simulink model "cddDcmApp"  (R2022b)
settings: Solver=FixedStepDiscrete  StartTime=0.0  StopTime=10.0  FixedStep=0.02  RelTol=1e-3
undefined symbols (not defined in the file or in its folder): CCPFaultType, DcChlType, HFAChlType, IcuChannelType, MtrRoutStaEnum, P_SignalPartType, P_Std_ReturnType, PowerChlType, SrsAdChlType, VGAChlType
(part 3 of 13)

== system cddDcmApp/DataServices_DID_D962_DID_D962_2_No_Of_Movements ==
  DataServices_DID_D962_DID_D962_2_No_Of_Movements_ReadData_sys : Subsystem
  DataServices_DID_D962_DID_D962_2_No_Of_Movements_WriteData_sys : Subsystem

== system cddDcmApp/DataServices_DID_D963_ComplexStructure_2 ==
  DataServices_DID_D963_ComplexStructure_2_ReadData_sys : Subsystem

== system cddDcmApp/DataServices_DID_D963_DID_D963_1_Requested_Position ==
  DataServices_DID_D963_DID_D963_1_Requested_Position_ReadData_sys : Subsystem

== system cddDcmApp/DataServices_DID_D963_DID_D963_2_Actual_Position ==
  DataServices_DID_D963_DID_D963_2_Actual_Position_ReadData_sys : Subsystem

== system cddDcmApp/DataServices_DID_D964_DID_D964_1_step_Range ==
  DataServices_DID_D964_DID_D964_1_step_Range_ReadData_sys : Subsystem
  DataServices_DID_D964_DID_D964_1_step_Range_WriteData_sys : Subsystem

== system cddDcmApp/DataServices_DID_D964_DID_D964_2_No_Of_Movements ==
  DataServices_DID_D964_DID_D964_2_No_Of_Movements_ReadData_sys : Subsystem
  DataServices_DID_D964_DID_D964_2_No_Of_Movements_WriteData_sys : Subsystem

== system cddDcmApp/DataServices_DID_D965_ComplexStructure_2 ==
  DataServices_DID_D965_ComplexStructure_2_ReadData_sys : Subsystem

== system cddDcmApp/DataServices_DID_D965_DID_D965_1_Requested_Position ==
  DataServices_DID_D965_DID_D965_1_Requested_Position_ReadData_sys : Subsystem

== system cddDcmApp/DataServices_DID_D965_DID_D965_2_Actual_Position ==
  DataServices_DID_D965_DID_D965_2_Actual_Position_ReadData_sys : Subsystem

== system cddDcmApp/DataServices_DID_D966_DID_D966_1_step_Range ==
  DataServices_DID_D966_DID_D966_1_step_Range_ReadData_sys : Subsystem
  DataServices_DID_D966_DID_D966_1_step_Range_WriteData_sys : Subsystem

== system cddDcmApp/DataServices_DID_D966_DID_D966_2_No_Of_Movements ==
  DataServices_DID_D966_DID_D966_2_No_Of_Movements_ReadData_sys : Subsystem
  DataServices_DID_D966_DID_D966_2_No_Of_Movements_WriteData_sys : Subsystem

== system cddDcmApp/DataServices_DID_D967_ComplexStructure_2 ==
  DataServices_DID_D967_ComplexStructure_2_ReadData_sys : Subsystem

== system cddDcmApp/DataServices_DID_D967_DID_D967_1_Requested_Position ==
  DataServices_DID_D967_DID_D967_1_Requested_Position_ReadData_sys : Subsystem

== system cddDcmApp/DataServices_DID_D967_DID_D967_2_Actual_Position ==
  DataServices_DID_D967_DID_D967_2_Actual_Position_ReadData_sys : Subsystem

== system cddDcmApp/DataServices_DID_D968_DID_D968_1_step_Range ==
  DataServices_DID_D968_DID_D968_1_step_Range_ReadData_sys : Subsystem
  DataServices_DID_D968_DID_D968_1_step_Range_WriteData_sys : Subsystem

== system cddDcmApp/DataServices_DID_D968_DID_D968_2_No_Of_Movements ==
  DataServices_DID_D968_DID_D968_2_No_Of_Movements_ReadData_sys : Subsystem
  DataServices_DID_D968_DID_D968_2_No_Of_Movements_WriteData_sys : Subsystem

== system cddDcmApp/DataServices_DID_D969_ComplexStructure_2 ==
  DataServices_DID_D969_ComplexStructure_2_ReadData_sys : Subsystem

== system cddDcmApp/DataServices_DID_D969_DID_D969_1_Requested_Position ==
  DataServices_DID_D969_DID_D969_1_Requested_Position_ReadData_sys : Subsystem

== system cddDcmApp/DataServices_DID_D969_DID_D969_2_Actual_Position ==
  DataServices_DID_D969_DID_D969_2_Actual_Position_ReadData_sys : Subsystem

== system cddDcmApp/DataServices_DID_D970_Stepper_Motor_12_Mode_Panel_First_Left_Flap_DID_D970_1_step_Range ==
  DataServices_DID_D970_Stepper_Motor_12_Mode_Panel_First_Left_Flap_DID_D970_1_step_Range_ConditionCheckRead_sys : Subsystem
  DataServices_DID_D970_Stepper_Motor_12_Mode_Panel_First_Left_Flap_DID_D970_1_step_Range_ReadData_sys : Subsystem
  DataServices_DID_D970_Stepper_Motor_12_Mode_Panel_First_Left_Flap_DID_D970_1_step_Range_WriteData_sys : Subsystem

== system cddDcmApp/DataServices_DID_D970_Stepper_Motor_12_Mode_Panel_First_Left_Flap_DID_D970_2_No_Of_Movements ==
  DataServices_DID_D970_Stepper_Motor_12_Mode_Panel_First_Left_Flap_DID_D970_2_No_Of_Movements_ConditionCheckRead_sys : Subsystem
  DataServices_DID_D970_Stepper_Motor_12_Mode_Panel_First_Left_Flap_DID_D970_2_No_Of_Movements_ReadData_sys : Subsystem
  DataServices_DID_D970_Stepper_Motor_12_Mode_Panel_First_Left_Flap_DID_D970_2_No_Of_Movements_WriteData_sys : Subsystem

== system cddDcmApp/DataServices_DID_D971_Stepper_Motor_12_Mode_Panel_First_Left_Flap_position_ComplexStructure_2 ==
  DataServices_DID_D971_Stepper_Motor_12_Mode_Panel_First_Left_Flap_position_ComplexStructure_2_ConditionCheckRead_sys : Subsystem
  DataServices_DID_D971_Stepper_Motor_12_Mode_Panel_First_Left_Flap_position_ComplexStructure_2_ReadData_sys : Subsystem

== system cddDcmApp/DataServices_DID_D971_Stepper_Motor_12_Mode_Panel_First_Left_Flap_position_DID_D971_1_Requested_Position ==
  DataServices_DID_D971_Stepper_Motor_12_Mode_Panel_First_Left_Flap_position_DID_D971_1_Requested_Position_ConditionCheckRead_sys : Subsystem
  DataServices_DID_D971_Stepper_Motor_12_Mode_Panel_First_Left_Flap_position_DID_D971_1_Requested_Position_ReadData_sys : Subsystem

== system cddDcmApp/DataServices_DID_D971_Stepper_Motor_12_Mode_Panel_First_Left_Flap_position_DID_D971_2_Actual_Position ==
  DataServices_DID_D971_Stepper_Motor_12_Mode_Panel_First_Left_Flap_position_DID_D971_2_Actual_Position_ConditionCheckRead_sys : Subsystem
  DataServices_DID_D971_Stepper_Motor_12_Mode_Panel_First_Left_Flap_position_DID_D971_2_Actual_Position_ReadData_sys : Subsystem

== system cddDcmApp/DataServices_DID_D972_Stepper_Motor_13_Mode_Panel_First_Side_Left_Flap_DID_D972_1_step_Range ==
  DataServices_DID_D972_Stepper_Motor_13_Mode_Panel_First_Side_Left_Flap_DID_D972_1_step_Range_ConditionCheckRead_sys : Subsystem
  DataServices_DID_D972_Stepper_Motor_13_Mode_Panel_First_Side_Left_Flap_DID_D972_1_step_Range_ReadData_sys : Subsystem
  DataServices_DID_D972_Stepper_Motor_13_Mode_Panel_First_Side_Left_Flap_DID_D972_1_step_Range_WriteData_sys : Subsystem

== system cddDcmApp/DataServices_DID_D972_Stepper_Motor_13_Mode_Panel_First_Side_Left_Flap_DID_D972_2_No_Of_Movements ==
  DataServices_DID_D972_Stepper_Motor_13_Mode_Panel_First_Side_Left_Flap_DID_D972_2_No_Of_Movements_ConditionCheckRead_sys : Subsystem
  DataServices_DID_D972_Stepper_Motor_13_Mode_Panel_First_Side_Left_Flap_DID_D972_2_No_Of_Movements_ReadData_sys : Subsystem
  DataServices_DID_D972_Stepper_Motor_13_Mode_Panel_First_Side_Left_Flap_DID_D972_2_No_Of_Movements_WriteData_sys : Subsystem

== system cddDcmApp/DataServices_DID_D973_Stepper_Motor_13_Mode_Panel_First_Side_Left_Flap_position_ComplexStructure_2 ==
  DataServices_DID_D973_Stepper_Motor_13_Mode_Panel_First_Side_Left_Flap_position_ComplexStructure_2_ConditionCheckRead_sys : Subsystem
  DataServices_DID_D973_Stepper_Motor_13_Mode_Panel_First_Side_Left_Flap_position_ComplexStructure_2_ReadData_sys : Subsystem

== system cddDcmApp/DataServices_DID_D973_Stepper_Motor_13_Mode_Panel_First_Side_Left_Flap_position_DID_D973_1_Requested_Position ==
  DataServices_DID_D973_Stepper_Motor_13_Mode_Panel_First_Side_Left_Flap_position_DID_D973_1_Requested_Position_ConditionCheckRead_sys : Subsystem
  DataServices_DID_D973_Stepper_Motor_13_Mode_Panel_First_Side_Left_Flap_position_DID_D973_1_Requested_Position_ReadData_sys : Subsystem

== system cddDcmApp/DataServices_DID_D973_Stepper_Motor_13_Mode_Panel_First_Side_Left_Flap_position_DID_D973_2_Actual_Position ==
  DataServices_DID_D973_Stepper_Motor_13_Mode_Panel_First_Side_Left_Flap_position_DID_D973_2_Actual_Position_ConditionCheckRead_sys : Subsystem
  DataServices_DID_D973_Stepper_Motor_13_Mode_Panel_First_Side_Left_Flap_position_DID_D973_2_Actual_Position_ReadData_sys : Subsystem

== system cddDcmApp/DataServices_DID_D974_Stepper_Motor_14_Mode_Panel_First_Right_Flap_DID_D974_1_step_Range ==
  DataServices_DID_D974_Stepper_Motor_14_Mode_Panel_First_Right_Flap_DID_D974_1_step_Range_ConditionCheckRead_sys : Subsystem
  DataServices_DID_D974_Stepper_Motor_14_Mode_Panel_First_Right_Flap_DID_D974_1_step_Range_ReadData_sys : Subsystem
  DataServices_DID_D974_Stepper_Motor_14_Mode_Panel_First_Right_Flap_DID_D974_1_step_Range_WriteData_sys : Subsystem

== system cddDcmApp/DataServices_DID_D974_Stepper_Motor_14_Mode_Panel_First_Right_Flap_DID_D974_2_No_Of_Movements ==
  DataServices_DID_D974_Stepper_Motor_14_Mode_Panel_First_Right_Flap_DID_D974_2_No_Of_Movements_ConditionCheckRead_sys : Subsystem
  DataServices_DID_D974_Stepper_Motor_14_Mode_Panel_First_Right_Flap_DID_D974_2_No_Of_Movements_ReadData_sys : Subsystem
  DataServices_DID_D974_Stepper_Motor_14_Mode_Panel_First_Right_Flap_DID_D974_2_No_Of_Movements_WriteData_sys : Subsystem

== system cddDcmApp/DataServices_DID_D975_Stepper_Motor_14_Mode_Panel_First_Right_Flap_position_ComplexStructure_2 ==
  DataServices_DID_D975_Stepper_Motor_14_Mode_Panel_First_Right_Flap_position_ComplexStructure_2_ConditionCheckRead_sys : Subsystem
  DataServices_DID_D975_Stepper_Motor_14_Mode_Panel_First_Right_Flap_position_ComplexStructure_2_ReadData_sys : Subsystem

== system cddDcmApp/DataServices_DID_D975_Stepper_Motor_14_Mode_Panel_First_Right_Flap_position_DID_D975_1_Requested_Position ==
  DataServices_DID_D975_Stepper_Motor_14_Mode_Panel_First_Right_Flap_position_DID_D975_1_Requested_Position_ConditionCheckRead_sys : Subsystem
  DataServices_DID_D975_Stepper_Motor_14_Mode_Panel_First_Right_Flap_position_DID_D975_1_Requested_Position_ReadData_sys : Subsystem

== system cddDcmApp/DataServices_DID_D975_Stepper_Motor_14_Mode_Panel_First_Right_Flap_position_DID_D975_2_Actual_Position ==
  DataServices_DID_D975_Stepper_Motor_14_Mode_Panel_First_Right_Flap_position_DID_D975_2_Actual_Position_ConditionCheckRead_sys : Subsystem
  DataServices_DID_D975_Stepper_Motor_14_Mode_Panel_First_Right_Flap_position_DID_D975_2_Actual_Position_ReadData_sys : Subsystem

== system cddDcmApp/DataServices_DID_D976_Stepper_Motor_15_Mode_Panel_First_Side_Right_Flap_DID_D976_1_step_Range ==
  DataServices_DID_D976_Stepper_Motor_15_Mode_Panel_First_Side_Right_Flap_DID_D976_1_step_Range_ConditionCheckRead_sys : Subsystem
  DataServices_DID_D976_Stepper_Motor_15_Mode_Panel_First_Side_Right_Flap_DID_D976_1_step_Range_ReadData_sys : Subsystem
  DataServices_DID_D976_Stepper_Motor_15_Mode_Panel_First_Side_Right_Flap_DID_D976_1_step_Range_WriteData_sys : Subsystem

== system cddDcmApp/DataServices_DID_D976_Stepper_Motor_15_Mode_Panel_First_Side_Right_Flap_DID_D976_2_No_Of_Movements ==
  DataServices_DID_D976_Stepper_Motor_15_Mode_Panel_First_Side_Right_Flap_DID_D976_2_No_Of_Movements_ConditionCheckRead_sys : Subsystem
  DataServices_DID_D976_Stepper_Motor_15_Mode_Panel_First_Side_Right_Flap_DID_D976_2_No_Of_Movements_ReadData_sys : Subsystem
  DataServices_DID_D976_Stepper_Motor_15_Mode_Panel_First_Side_Right_Flap_DID_D976_2_No_Of_Movements_WriteData_sys : Subsystem

== system cddDcmApp/DataServices_DID_D977_Stepper_Motor_15_Mode_Panel_First_Side_Right_Flap_position_ComplexStructure_2 ==
  DataServices_DID_D977_Stepper_Motor_15_Mode_Panel_First_Side_Right_Flap_position_ComplexStructure_2_ConditionCheckRead_sys : Subsystem
  DataServices_DID_D977_Stepper_Motor_15_Mode_Panel_First_Side_Right_Flap_position_ComplexStructure_2_ReadData_sys : Subsystem

== system cddDcmApp/DataServices_DID_D977_Stepper_Motor_15_Mode_Panel_First_Side_Right_Flap_position_DID_D977_1_Requested_Position ==
  DataServices_DID_D977_Stepper_Motor_15_Mode_Panel_First_Side_Right_Flap_position_DID_D977_1_Requested_Position_ReadData_sys : Subsystem

== system cddDcmApp/DataServices_DID_D977_Stepper_Motor_15_Mode_Panel_First_Side_Right_Flap_position_DID_D977_2_Actual_Position ==
  DataServices_DID_D977_Stepper_Motor_15_Mode_Panel_First_Side_Right_Flap_position_DID_D977_2_Actual_Position_ConditionCheckRead_sys : Subsystem
  DataServices_DID_D977_Stepper_Motor_15_Mode_Panel_First_Side_Right_Flap_position_DID_D977_2_Actual_Position_ReadData_sys : Subsystem

== system cddDcmApp/DataServices_DID_D978_Stepper_Motor_16_Mode_Floor_First_Left_Flap_DID_D978_1_step_Range ==
  DataServices_DID_D978_Stepper_Motor_16_Mode_Floor_First_Left_Flap_DID_D978_1_step_Range_ConditionCheckRead_sys : Subsystem
  DataServices_DID_D978_Stepper_Motor_16_Mode_Floor_First_Left_Flap_DID_D978_1_step_Range_ReadData_sys : Subsystem
  DataServices_DID_D978_Stepper_Motor_16_Mode_Floor_First_Left_Flap_DID_D978_1_step_Range_WriteData_sys : Subsystem

== system cddDcmApp/DataServices_DID_D978_Stepper_Motor_16_Mode_Floor_First_Left_Flap_DID_D978_2_No_Of_Movements ==
  DataServices_DID_D978_Stepper_Motor_16_Mode_Floor_First_Left_Flap_DID_D978_2_No_Of_Movements_ConditionCheckRead_sys : Subsystem
  DataServices_DID_D978_Stepper_Motor_16_Mode_Floor_First_Left_Flap_DID_D978_2_No_Of_Movements_ReadData_sys : Subsystem
  DataServices_DID_D978_Stepper_Motor_16_Mode_Floor_First_Left_Flap_DID_D978_2_No_Of_Movements_WriteData_sys : Subsystem

== system cddDcmApp/DataServices_DID_D979_Stepper_Motor_16_Mode_Floor_First_Left_Flap_position_ComplexStructure_2 ==
  DataServices_DID_D979_Stepper_Motor_16_Mode_Floor_First_Left_Flap_position_ComplexStructure_2_ConditionCheckRead_sys : Subsystem
  DataServices_DID_D979_Stepper_Motor_16_Mode_Floor_First_Left_Flap_position_ComplexStructure_2_ReadData_sys : Subsystem

== system cddDcmApp/DataServices_DID_D979_Stepper_Motor_16_Mode_Floor_First_Left_Flap_position_DID_D979_1_Requested_Position ==
  DataServices_DID_D979_Stepper_Motor_16_Mode_Floor_First_Left_Flap_position_DID_D979_1_Requested_Position_ConditionCheckRead_sys : Subsystem
  DataServices_DID_D979_Stepper_Motor_16_Mode_Floor_First_Left_Flap_position_DID_D979_1_Requested_Position_ReadData_sys : Subsystem

== system cddDcmApp/DataServices_DID_D979_Stepper_Motor_16_Mode_Floor_First_Left_Flap_position_DID_D979_2_Actual_Position ==
  DataServices_DID_D979_Stepper_Motor_16_Mode_Floor_First_Left_Flap_position_DID_D979_2_Actual_Position_ConditionCheckRead_sys : Subsystem
  DataServices_DID_D979_Stepper_Motor_16_Mode_Floor_First_Left_Flap_position_DID_D979_2_Actual_Position_ReadData_sys : Subsystem

== system cddDcmApp/DataServices_DID_D97A_Stepper_Motor_17_Mode_Floor_First_Right_Flap_DID_D97A_1_step_Range ==
  DataServices_DID_D97A_Stepper_Motor_17_Mode_Floor_First_Right_Flap_DID_D97A_1_step_Range_ConditionCheckRead_sys : Subsystem
  DataServices_DID_D97A_Stepper_Motor_17_Mode_Floor_First_Right_Flap_DID_D97A_1_step_Range_ReadData_sys : Subsystem
  DataServices_DID_D97A_Stepper_Motor_17_Mode_Floor_First_Right_Flap_DID_D97A_1_step_Range_WriteData_sys : Subsystem

== system cddDcmApp/DataServices_DID_D97A_Stepper_Motor_17_Mode_Floor_First_Right_Flap_DID_D97A_2_No_Of_Movements ==
  DataServices_DID_D97A_Stepper_Motor_17_Mode_Floor_First_Right_Flap_DID_D97A_2_No_Of_Movements_ConditionCheckRead_sys : Subsystem
  DataServices_DID_D97A_Stepper_Motor_17_Mode_Floor_First_Right_Flap_DID_D97A_2_No_Of_Movements_ReadData_sys : Subsystem
  DataServices_DID_D97A_Stepper_Motor_17_Mode_Floor_First_Right_Flap_DID_D97A_2_No_Of_Movements_WriteData_sys : Subsystem

== system cddDcmApp/DataServices_DID_D97B_Stepper_Motor_17_Mode_Floor_First_Right_Flap_position_ComplexStructure_2 ==
  DataServices_DID_D97B_Stepper_Motor_17_Mode_Floor_First_Right_Flap_position_ComplexStructure_2_ConditionCheckRead_sys : Subsystem
  DataServices_DID_D97B_Stepper_Motor_17_Mode_Floor_First_Right_Flap_position_ComplexStructure_2_ReadData_sys : Subsystem

== system cddDcmApp/DataServices_DID_D97B_Stepper_Motor_17_Mode_Floor_First_Right_Flap_position_DID_D97B_1_Requested_Position ==
  DataServices_DID_D97B_Stepper_Motor_17_Mode_Floor_First_Right_Flap_position_DID_D97B_1_Requested_Position_ConditionCheckRead_sys : Subsystem
  DataServices_DID_D97B_Stepper_Motor_17_Mode_Floor_First_Right_Flap_position_DID_D97B_1_Requested_Position_ReadData_sys : Subsystem

== system cddDcmApp/DataServices_DID_D97B_Stepper_Motor_17_Mode_Floor_First_Right_Flap_position_DID_D97B_2_Actual_Position ==
  DataServices_DID_D97B_Stepper_Motor_17_Mode_Floor_First_Right_Flap_position_DID_D97B_2_Actual_Position_ConditionCheckRead_sys : Subsystem
  DataServices_DID_D97B_Stepper_Motor_17_Mode_Floor_First_Right_Flap_position_DID_D97B_2_Actual_Position_ReadData_sys : Subsystem

== system cddDcmApp/DataServices_DID_D97C_Stepper_Motor_18_Mode_PanelFloor_Second_Left_Flap_DID_D97C_1_step_Range ==
  DataServices_DID_D97C_Stepper_Motor_18_Mode_PanelFloor_Second_Left_Flap_DID_D97C_1_step_Range_ConditionCheckRead_sys : Subsystem
  DataServices_DID_D97C_Stepper_Motor_18_Mode_PanelFloor_Second_Left_Flap_DID_D97C_1_step_Range_ReadData_sys : Subsystem
  DataServices_DID_D97C_Stepper_Motor_18_Mode_PanelFloor_Second_Left_Flap_DID_D97C_1_step_Range_WriteData_sys : Subsystem

== system cddDcmApp/DataServices_DID_D97C_Stepper_Motor_18_Mode_PanelFloor_Second_Left_Flap_DID_D97C_2_No_Of_Movements ==
  DataServices_DID_D97C_Stepper_Motor_18_Mode_PanelFloor_Second_Left_Flap_DID_D97C_2_No_Of_Movements_ConditionCheckRead_sys : Subsystem
  DataServices_DID_D97C_Stepper_Motor_18_Mode_PanelFloor_Second_Left_Flap_DID_D97C_2_No_Of_Movements_ReadData_sys : Subsystem
  DataServices_DID_D97C_Stepper_Motor_18_Mode_PanelFloor_Second_Left_Flap_DID_D97C_2_No_Of_Movements_WriteData_sys : Subsystem

== system cddDcmApp/DataServices_DID_D97D_Stepper_Motor_18_Mode_PanelFloor_Second_Left_Flap_position_ComplexStructure_2 ==
  DataServices_DID_D97D_Stepper_Motor_18_Mode_PanelFloor_Second_Left_Flap_position_ComplexStructure_2_ConditionCheckRead_sys : Subsystem
  DataServices_DID_D97D_Stepper_Motor_18_Mode_PanelFloor_Second_Left_Flap_position_ComplexStructure_2_ReadData_sys : Subsystem

== system cddDcmApp/DataServices_DID_D97D_Stepper_Motor_18_Mode_PanelFloor_Second_Left_Flap_position_DID_D97D_1_Requested_Position ==
  DataServices_DID_D97D_Stepper_Motor_18_Mode_PanelFloor_Second_Left_Flap_position_DID_D97D_1_Requested_Position_ReadData_sys : Subsystem

== system cddDcmApp/DataServices_DID_D97D_Stepper_Motor_18_Mode_PanelFloor_Second_Left_Flap_position_DID_D97D_2_Actual_Position ==
  DataServices_DID_D97D_Stepper_Motor_18_Mode_PanelFloor_Second_Left_Flap_position_DID_D97D_2_Actual_Position_ConditionCheckRead_sys : Subsystem
  DataServices_DID_D97D_Stepper_Motor_18_Mode_PanelFloor_Second_Left_Flap_position_DID_D97D_2_Actual_Position_ReadData_sys : Subsystem

== system cddDcmApp/DataServices_DID_D97E_Stepper_Motor_19_Mode_PanelFloor_Second_Right_Flap_DID_D97E_1_step_Range ==
  DataServices_DID_D97E_Stepper_Motor_19_Mode_PanelFloor_Second_Right_Flap_DID_D97E_1_step_Range_ConditionCheckRead_sys : Subsystem
  DataServices_DID_D97E_Stepper_Motor_19_Mode_PanelFloor_Second_Right_Flap_DID_D97E_1_step_Range_ReadData_sys : Subsystem
  DataServices_DID_D97E_Stepper_Motor_19_Mode_PanelFloor_Second_Right_Flap_DID_D97E_1_step_Range_WriteData_sys : Subsystem

== system cddDcmApp/DataServices_DID_D97E_Stepper_Motor_19_Mode_PanelFloor_Second_Right_Flap_DID_D97E_2_No_Of_Movements ==
  DataServices_DID_D97E_Stepper_Motor_19_Mode_PanelFloor_Second_Right_Flap_DID_D97E_2_No_Of_Movements_ConditionCheckRead_sys : Subsystem
  DataServices_DID_D97E_Stepper_Motor_19_Mode_PanelFloor_Second_Right_Flap_DID_D97E_2_No_Of_Movements_ReadData_sys : Subsystem
  DataServices_DID_D97E_Stepper_Motor_19_Mode_PanelFloor_Second_Right_Flap_DID_D97E_2_No_Of_Movements_WriteData_sys : Subsystem

== system cddDcmApp/DataServices_DID_D97F_Stepper_Motor_19_Mode_PanelFloor_Second_Right_Flap_position_ComplexStructure_2 ==
  DataServices_DID_D97F_Stepper_Motor_19_Mode_PanelFloor_Second_Right_Flap_position_ComplexStructure_2_ConditionCheckRead_sys : Subsystem
  DataServices_DID_D97F_Stepper_Motor_19_Mode_PanelFloor_Second_Right_Flap_position_ComplexStructure_2_ReadData_sys : Subsystem

== system cddDcmApp/DataServices_DID_D97F_Stepper_Motor_19_Mode_PanelFloor_Second_Right_Flap_position_DID_D97F_1_Requested_Position ==
  DataServices_DID_D97F_Stepper_Motor_19_Mode_PanelFloor_Second_Right_Flap_position_DID_D97F_1_Requested_Position_ReadData_sys : Subsystem

== system cddDcmApp/DataServices_DID_D97F_Stepper_Motor_19_Mode_PanelFloor_Second_Right_Flap_position_DID_D97F_2_Actual_Position ==
  DataServices_DID_D97F_Stepper_Motor_19_Mode_PanelFloor_Second_Right_Flap_position_DID_D97F_2_Actual_Position_ConditionCheckRead_sys : Subsystem
  DataServices_DID_D97F_Stepper_Motor_19_Mode_PanelFloor_Second_Right_Flap_position_DID_D97F_2_Actual_Position_ReadData_sys : Subsystem

== system cddDcmApp/DataServices_DID_D980_Vent_Grill_Actuator9_Second_Left_X_Flap_DID_D980 ==
  DataServices_DID_D980_Vent_Grill_Actuator9_Second_Left_X_Flap_DID_D980_ConditionCheckRead_sys : Subsystem
  DataServices_DID_D980_Vent_Grill_Actuator9_Second_Left_X_Flap_DID_D980_ReadData_sys : Subsystem
  DataServices_DID_D980_Vent_Grill_Actuator9_Second_Left_X_Flap_DID_D980_WriteData_sys : Subsystem

== system cddDcmApp/DataServices_DID_D980_Vent_Grill_Actuator9_Second_Left_X_Flap_DID_D980_2_No_Of_Movements ==
  DataServices_DID_D980_Vent_Grill_Actuator9_Second_Left_X_Flap_DID_D980_2_No_Of_Movements_ConditionCheckRead_sys : Subsystem
  DataServices_DID_D980_Vent_Grill_Actuator9_Second_Left_X_Flap_DID_D980_2_No_Of_Movements_ReadData_sys : Subsystem
  DataServices_DID_D980_Vent_Grill_Actuator9_Second_Left_X_Flap_DID_D980_2_No_Of_Movements_WriteData_sys : Subsystem

== system cddDcmApp/DataServices_DID_D981_Vent_Grill_Actuator9_Second_Left_X_Flap_position_ComplexStructure_2 ==
  DataServices_DID_D981_Vent_Grill_Actuator9_Second_Left_X_Flap_position_ComplexStructure_2_ConditionCheckRead_sys : Subsystem
  DataServices_DID_D981_Vent_Grill_Actuator9_Second_Left_X_Flap_position_ComplexStructure_2_ReadData_sys : Subsystem

== system cddDcmApp/DataServices_DID_D981_Vent_Grill_Actuator9_Second_Left_X_Flap_position_DID_D981_1_Requested_Position ==
  DataServices_DID_D981_Vent_Grill_Actuator9_Second_Left_X_Flap_position_DID_D981_1_Requested_Position_ConditionCheckRead_sys : Subsystem
  DataServices_DID_D981_Vent_Grill_Actuator9_Second_Left_X_Flap_position_DID_D981_1_Requested_Position_ReadData_sys : Subsystem

== system cddDcmApp/DataServices_DID_D981_Vent_Grill_Actuator9_Second_Left_X_Flap_position_DID_D981_2_Actual_Position ==
  DataServices_DID_D981_Vent_Grill_Actuator9_Second_Left_X_Flap_position_DID_D981_2_Actual_Position_ConditionCheckRead_sys : Subsystem
  DataServices_DID_D981_Vent_Grill_Actuator9_Second_Left_X_Flap_position_DID_D981_2_Actual_Position_ReadData_sys : Subsystem

== system cddDcmApp/DataServices_DID_D982_Vent_Grill_Actuator10_Second_Left_Y_Flap_DID_D982_1_step_Range ==
  DataServices_DID_D982_Vent_Grill_Actuator10_Second_Left_Y_Flap_DID_D982_1_step_Range_ConditionCheckRead_sys : Subsystem
  DataServices_DID_D982_Vent_Grill_Actuator10_Second_Left_Y_Flap_DID_D982_1_step_Range_ReadData_sys : Subsystem
  DataServices_DID_D982_Vent_Grill_Actuator10_Second_Left_Y_Flap_DID_D982_1_step_Range_WriteData_sys : Subsystem

== system cddDcmApp/DataServices_DID_D982_Vent_Grill_Actuator10_Second_Left_Y_Flap_DID_D982_2_No_Of_Movements ==
  DataServices_DID_D982_Vent_Grill_Actuator10_Second_Left_Y_Flap_DID_D982_2_No_Of_Movements_ConditionCheckRead_sys : Subsystem
  DataServices_DID_D982_Vent_Grill_Actuator10_Second_Left_Y_Flap_DID_D982_2_No_Of_Movements_ReadData_sys : Subsystem
  DataServices_DID_D982_Vent_Grill_Actuator10_Second_Left_Y_Flap_DID_D982_2_No_Of_Movements_WriteData_sys : Subsystem

== system cddDcmApp/DataServices_DID_D983_Vent_Grill_Actuator10_Second_Left_Y_Flap_position_ComplexStructure_2 ==
  DataServices_DID_D983_Vent_Grill_Actuator10_Second_Left_Y_Flap_position_ComplexStructure_2_ConditionCheckRead_sys : Subsystem
  DataServices_DID_D983_Vent_Grill_Actuator10_Second_Left_Y_Flap_position_ComplexStructure_2_ReadData_sys : Subsystem

== system cddDcmApp/DataServices_DID_D983_Vent_Grill_Actuator10_Second_Left_Y_Flap_position_DID_D983_1_Requested_Position ==
  DataServices_DID_D983_Vent_Grill_Actuator10_Second_Left_Y_Flap_position_DID_D983_1_Requested_Position_ConditionCheckRead_sys : Subsystem
  DataServices_DID_D983_Vent_Grill_Actuator10_Second_Left_Y_Flap_position_DID_D983_1_Requested_Position_ReadData_sys : Subsystem

== system cddDcmApp/DataServices_DID_D983_Vent_Grill_Actuator10_Second_Left_Y_Flap_position_DID_D983_2_Actual_Position ==
  DataServices_DID_D983_Vent_Grill_Actuator10_Second_Left_Y_Flap_position_DID_D983_2_Actual_Position_ConditionCheckRead_sys : Subsystem
  DataServices_DID_D983_Vent_Grill_Actuator10_Second_Left_Y_Flap_position_DID_D983_2_Actual_Position_ReadData_sys : Subsystem

== system cddDcmApp/DataServices_DID_D984_Vent_Grill_Actuator11_Second_Right_X_Flap_DID_D984_1_step_Range ==
  DataServices_DID_D984_Vent_Grill_Actuator11_Second_Right_X_Flap_DID_D984_1_step_Range_ConditionCheckRead_sys : Subsystem
  DataServices_DID_D984_Vent_Grill_Actuator11_Second_Right_X_Flap_DID_D984_1_step_Range_ReadData_sys : Subsystem
  DataServices_DID_D984_Vent_Grill_Actuator11_Second_Right_X_Flap_DID_D984_1_step_Range_WriteData_sys : Subsystem

== system cddDcmApp/DataServices_DID_D984_Vent_Grill_Actuator11_Second_Right_X_Flap_DID_D984_2_No_Of_Movements ==
  DataServices_DID_D984_Vent_Grill_Actuator11_Second_Right_X_Flap_DID_D984_2_No_Of_Movements_ConditionCheckRead_sys : Subsystem
  DataServices_DID_D984_Vent_Grill_Actuator11_Second_Right_X_Flap_DID_D984_2_No_Of_Movements_ReadData_sys : Subsystem
  DataServices_DID_D984_Vent_Grill_Actuator11_Second_Right_X_Flap_DID_D984_2_No_Of_Movements_WriteData_sys : Subsystem

== system cddDcmApp/DataServices_DID_D985_Vent_Grill_Actuator11_Second_Right_X_Flap_position_ComplexStructure_2 ==
  DataServices_DID_D985_Vent_Grill_Actuator11_Second_Right_X_Flap_position_ComplexStructure_2_ConditionCheckRead_sys : Subsystem
  DataServices_DID_D985_Vent_Grill_Actuator11_Second_Right_X_Flap_position_ComplexStructure_2_ReadData_sys : Subsystem

== system cddDcmApp/DataServices_DID_D985_Vent_Grill_Actuator11_Second_Right_X_Flap_position_DID_D985_1_Requested_Position ==
  DataServices_DID_D985_Vent_Grill_Actuator11_Second_Right_X_Flap_position_DID_D985_1_Requested_Position_ConditionCheckRead_sys : Subsystem
  DataServices_DID_D985_Vent_Grill_Actuator11_Second_Right_X_Flap_position_DID_D985_1_Requested_Position_ReadData_sys : Subsystem

== system cddDcmApp/DataServices_DID_D985_Vent_Grill_Actuator11_Second_Right_X_Flap_position_DID_D985_2_Actual_Position ==
  DataServices_DID_D985_Vent_Grill_Actuator11_Second_Right_X_Flap_position_DID_D985_2_Actual_Position_ConditionCheckRead_sys : Subsystem
  DataServices_DID_D985_Vent_Grill_Actuator11_Second_Right_X_Flap_position_DID_D985_2_Actual_Position_ReadData_sys : Subsystem

== system cddDcmApp/DataServices_DID_D986_Vent_Grill_Actuator12_Second_Right_Y_Flap_DID_D986_1_step_Range ==
  DataServices_DID_D986_Vent_Grill_Actuator12_Second_Right_Y_Flap_DID_D986_1_step_Range_ConditionCheckRead_sys : Subsystem
  DataServices_DID_D986_Vent_Grill_Actuator12_Second_Right_Y_Flap_DID_D986_1_step_Range_ReadData_sys : Subsystem
  DataServices_DID_D986_Vent_Grill_Actuator12_Second_Right_Y_Flap_DID_D986_1_step_Range_WriteData_sys : Subsystem

== system cddDcmApp/DataServices_DID_D986_Vent_Grill_Actuator12_Second_Right_Y_Flap_DID_D986_2_No_Of_Movements ==
  DataServices_DID_D986_Vent_Grill_Actuator12_Second_Right_Y_Flap_DID_D986_2_No_Of_Movements_ConditionCheckRead_sys : Subsystem
  DataServices_DID_D986_Vent_Grill_Actuator12_Second_Right_Y_Flap_DID_D986_2_No_Of_Movements_ReadData_sys : Subsystem
  DataServices_DID_D986_Vent_Grill_Actuator12_Second_Right_Y_Flap_DID_D986_2_No_Of_Movements_WriteData_sys : Subsystem

== system cddDcmApp/DataServices_DID_D987_Vent_Grill_Actuator12_Second_Right_Y_Flap_position_ComplexStructure_2 ==
  DataServices_DID_D987_Vent_Grill_Actuator12_Second_Right_Y_Flap_position_ComplexStructure_2_ConditionCheckRead_sys : Subsystem
  DataServices_DID_D987_Vent_Grill_Actuator12_Second_Right_Y_Flap_position_ComplexStructure_2_ReadData_sys : Subsystem

== system cddDcmApp/DataServices_DID_D987_Vent_Grill_Actuator12_Second_Right_Y_Flap_position_DID_D987_1_Requested_Position ==
  DataServices_DID_D987_Vent_Grill_Actuator12_Second_Right_Y_Flap_position_DID_D987_1_Requested_Position_ConditionCheckRead_sys : Subsystem
  DataServices_DID_D987_Vent_Grill_Actuator12_Second_Right_Y_Flap_position_DID_D987_1_Requested_Position_ReadData_sys : Subsystem

== system cddDcmApp/DataServices_DID_D987_Vent_Grill_Actuator12_Second_Right_Y_Flap_position_DID_D987_2_Actual_Position ==
  DataServices_DID_D987_Vent_Grill_Actuator12_Second_Right_Y_Flap_position_DID_D987_2_Actual_Position_ConditionCheckRead_sys : Subsystem
  DataServices_DID_D987_Vent_Grill_Actuator12_Second_Right_Y_Flap_position_DID_D987_2_Actual_Position_ReadData_sys : Subsystem

== system cddDcmApp/DataServices_DID_D988_Vent_Grill_Actuator13_Second_Right_Y_Flap_DID_D988_1_step_Range ==
  DataServices_DID_D988_Vent_Grill_Actuator13_Second_Right_Y_Flap_DID_D988_1_step_Range_ReadData_sys : Subsystem
  DataServices_DID_D988_Vent_Grill_Actuator13_Second_Right_Y_Flap_DID_D988_1_step_Range_WriteData_sys : Subsystem

== system cddDcmApp/DataServices_DID_D988_Vent_Grill_Actuator13_Second_Right_Y_Flap_DID_D988_2_No_Of_Movements ==
  DataServices_DID_D988_Vent_Grill_Actuator13_Second_Right_Y_Flap_DID_D988_2_No_Of_Movements_ReadData_sys : Subsystem
  DataServices_DID_D988_Vent_Grill_Actuator13_Second_Right_Y_Flap_DID_D988_2_No_Of_Movements_WriteData_sys : Subsystem

== system cddDcmApp/DataServices_DID_D989_Vent_Grill_Actuator13_Second_Right_Y_Flap_position_DID_D989_1_Requested_Position ==
  DataServices_DID_D989_Vent_Grill_Actuator13_Second_Right_Y_Flap_position_DID_D989_1_Requested_Position_ReadData_sys : Subsystem

== system cddDcmApp/DataServices_DID_D989_Vent_Grill_Actuator13_Second_Right_Y_Flap_position_DID_D989_2_Actual_Position ==
  DataServices_DID_D989_Vent_Grill_Actuator13_Second_Right_Y_Flap_position_DID_D989_2_Actual_Position_ReadData_sys : Subsystem

== system cddDcmApp/DataServices_DID_D989_Vent_Grill_Actuator13_Second_Right_Y_Flap_position_DID_D989_3_Calibration_status ==
  DataServices_DID_D989_Vent_Grill_Actuator13_Second_Right_Y_Flap_position_DID_D989_3_Calibration_status_ReadData_sys : Subsystem

== system cddDcmApp/DataServices_DID_D989_Vent_Grill_Actuator13_Second_Right_Y_Flap_position_DID_D989_4_Working_Status ==
  DataServices_DID_D989_Vent_Grill_Actuator13_Second_Right_Y_Flap_position_DID_D989_4_Working_Status_ReadData_sys : Subsystem

== system cddDcmApp/DataServices_DID_D98A_Vent_Grill_Actuator14_Second_Right_Y_Flap_DID_D98A_1_step_Range ==
  DataServices_DID_D98A_Vent_Grill_Actuator14_Second_Right_Y_Flap_DID_D98A_1_step_Range_ReadData_sys : Subsystem
  DataServices_DID_D98A_Vent_Grill_Actuator14_Second_Right_Y_Flap_DID_D98A_1_step_Range_WriteData_sys : Subsystem

== system cddDcmApp/DataServices_DID_D98A_Vent_Grill_Actuator14_Second_Right_Y_Flap_DID_D98A_2_No_Of_Movements ==
  DataServices_DID_D98A_Vent_Grill_Actuator14_Second_Right_Y_Flap_DID_D98A_2_No_Of_Movements_ReadData_sys : Subsystem
  DataServices_DID_D98A_Vent_Grill_Actuator14_Second_Right_Y_Flap_DID_D98A_2_No_Of_Movements_WriteData_sys : Subsystem

== system cddDcmApp/DataServices_DID_D98B_Vent_Grill_Actuator14_Second_Right_Y_Flap_position_DID_D98B_1_Requested_Position ==
  DataServices_DID_D98B_Vent_Grill_Actuator14_Second_Right_Y_Flap_position_DID_D98B_1_Requested_Position_ReadData_sys : Subsystem

== system cddDcmApp/DataServices_DID_D98B_Vent_Grill_Actuator14_Second_Right_Y_Flap_position_DID_D98B_2_Actual_Position ==
  DataServices_DID_D98B_Vent_Grill_Actuator14_Second_Right_Y_Flap_position_DID_D98B_2_Actual_Position_ReadData_sys : Subsystem

== system cddDcmApp/DataServices_DID_D98B_Vent_Grill_Actuator14_Second_Right_Y_Flap_position_DID_D98B_3_Calibration_Status ==
  DataServices_DID_D98B_Vent_Grill_Actuator14_Second_Right_Y_Flap_position_DID_D98B_3_Calibration_Status_ReadData_sys : Subsystem

== system cddDcmApp/DataServices_DID_D98B_Vent_Grill_Actuator14_Second_Right_Y_Flap_position_DID_D98B_4_Working_Status ==
  DataServices_DID_D98B_Vent_Grill_Actuator14_Second_Right_Y_Flap_position_DID_D98B_4_Working_Status_ReadData_sys : Subsystem

== system cddDcmApp/DataServices_DID_D98C_Vent_Grill_Actuator15_Second_Right_Y_Flap_DID_D98C_1_step_Range ==
  DataServices_DID_D98C_Vent_Grill_Actuator15_Second_Right_Y_Flap_DID_D98C_1_step_Range_ReadData_sys : Subsystem
  DataServices_DID_D98C_Vent_Grill_Actuator15_Second_Right_Y_Flap_DID_D98C_1_step_Range_WriteData_sys : Subsystem

== system cddDcmApp/DataServices_DID_D98C_Vent_Grill_Actuator15_Second_Right_Y_Flap_DID_D98C_2_No_Of_Movements ==
  DataServices_DID_D98C_Vent_Grill_Actuator15_Second_Right_Y_Flap_DID_D98C_2_No_Of_Movements_ReadData_sys : Subsystem
  DataServices_DID_D98C_Vent_Grill_Actuator15_Second_Right_Y_Flap_DID_D98C_2_No_Of_Movements_WriteData_sys : Subsystem

== system cddDcmApp/DataServices_DID_D98D_Vent_Grill_Actuator15_Second_Right_Y_Flap_position_ComplexStructure_2 ==
  DataServices_DID_D98D_Vent_Grill_Actuator15_Second_Right_Y_Flap_position_ComplexStructure_2_ReadData_sys : Subsystem

== system cddDcmApp/DataServices_DID_D98D_Vent_Grill_Actuator15_Second_Right_Y_Flap_position_DID_D98D_1_Requested_Position ==
  DataServices_DID_D98D_Vent_Grill_Actuator15_Second_Right_Y_Flap_position_DID_D98D_1_Requested_Position_ReadData_sys : Subsystem

== system cddDcmApp/DataServices_DID_D98D_Vent_Grill_Actuator15_Second_Right_Y_Flap_position_DID_D98D_2_Actual_Position ==
  DataServices_DID_D98D_Vent_Grill_Actuator15_Second_Right_Y_Flap_position_DID_D98D_2_Actual_Position_ReadData_sys : Subsystem

== system cddDcmApp/DataServices_DID_D98E_Vent_Grill_Actuator16_Second_Right_Y_Flap_DID_D98E_1_step_Range ==
  DataServices_DID_D98E_Vent_Grill_Actuator16_Second_Right_Y_Flap_DID_D98E_1_step_Range_ReadData_sys : Subsystem
  DataServices_DID_D98E_Vent_Grill_Actuator16_Second_Right_Y_Flap_DID_D98E_1_step_Range_WriteData_sys : Subsystem

== system cddDcmApp/DataServices_DID_D98E_Vent_Grill_Actuator16_Second_Right_Y_Flap_DID_D98E_2_No_Of_Movements ==
  DataServices_DID_D98E_Vent_Grill_Actuator16_Second_Right_Y_Flap_DID_D98E_2_No_Of_Movements_ReadData_sys : Subsystem
  DataServices_DID_D98E_Vent_Grill_Actuator16_Second_Right_Y_Flap_DID_D98E_2_No_Of_Movements_WriteData_sys : Subsystem

== system cddDcmApp/DataServices_DID_D98F_Vent_Grill_Actuator16_Second_Right_Y_Flap_position_ComplexStructure_2 ==
  DataServices_DID_D98F_Vent_Grill_Actuator16_Second_Right_Y_Flap_position_ComplexStructure_2_ReadData_sys : Subsystem

== system cddDcmApp/DataServices_DID_D98F_Vent_Grill_Actuator16_Second_Right_Y_Flap_position_DID_D98F_1_Requested_Position ==
  DataServices_DID_D98F_Vent_Grill_Actuator16_Second_Right_Y_Flap_position_DID_D98F_1_Requested_Position_ReadData_sys : Subsystem

== system cddDcmApp/DataServices_DID_D98F_Vent_Grill_Actuator16_Second_Right_Y_Flap_position_DID_D98F_2_Actual_Position ==
  DataServices_DID_D98F_Vent_Grill_Actuator16_Second_Right_Y_Flap_position_DID_D98F_2_Actual_Position_ReadData_sys : Subsystem

== system cddDcmApp/DataServices_DID_DA00_DID_DA00_Read_CCM_Internal_Data_For ==
  DataServices_DID_DA00_DID_DA00_Read_CCM_Internal_Data_For_Analysis_sys : Subsystem

== system cddDcmApp/Dcm_Reset_Hard_sys ==
  function : TriggerPort  TriggerType=function-call  VariantControl=(inherit)

== system cddDcmApp/Dcm_Reset_JumpToBootLoader_sys ==
  function : TriggerPort  TriggerType=function-call  VariantControl=(inherit)

== system cddDcmApp/DiagSessionCtrl_Default_sys ==
  function : TriggerPort  TriggerType=function-call  VariantControl=(inherit)

== system cddDcmApp/RoutineServices_RID_DF20_Run_Calibration_on_All_Damper_Motors ==
  RoutineServices_RID_DF20_Run_Calibration_on_All_Damper_Motors_RequestResults_sys : Subsystem
  RoutineServices_RID_DF20_Run_Calibration_on_All_Damper_Motors_Start_sys : Subsystem

== system cddDcmApp/RoutineServices_RID_DF40_HFA_motor_auto_addressing ==
  RoutineServices_RID_DF40_HFA_motor_auto_addressing_RequestResults_sys : Subsystem
  RoutineServices_RID_DF40_HFA_motor_auto_addressing_Start_sys : Subsystem
